simulink subsystem "ButtonStatesRF"  (R2023b)
settings: Solver=VariableStepAuto  StartTime=0.0  StopTime=10.0  RelTol=1e-3
undefined symbols (not defined in the file or in its folder): eStateName
libraries: simulink x5
(part 1 of 2)
settings Model: DataDictionary=ButtonLogicData.sldd  EnableAccessToBaseWorkspace=off  Creator=pkpam  LastModifiedBy=pkpam

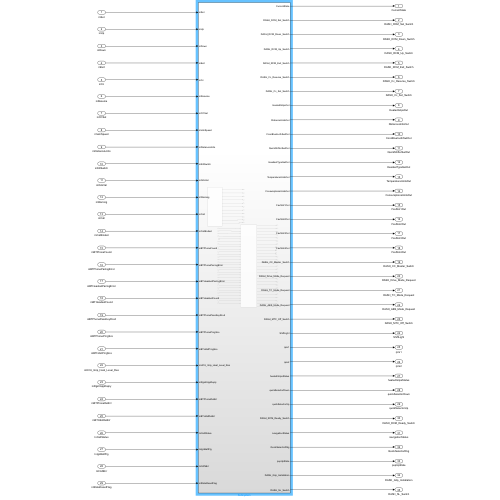
[thumbnail: Simulink's stored preview of the top-level diagram]
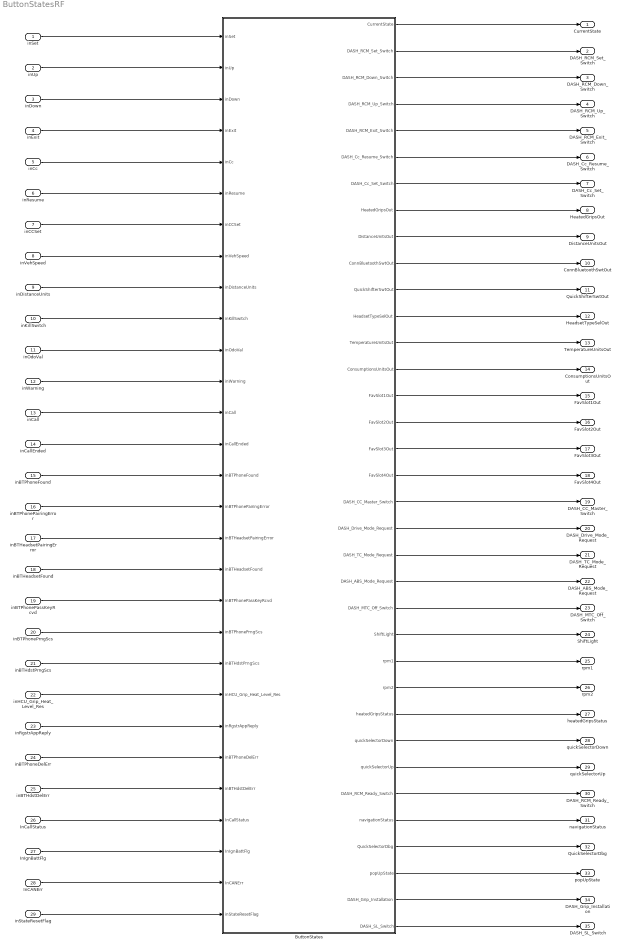
== system ButtonStatesRF ==
  inSet : Inport  OutDataTypeStr=boolean
  inUp : Inport  Port=2  OutDataTypeStr=boolean
  inDown : Inport  Port=3  OutDataTypeStr=boolean
  inExit : Inport  Port=4  OutDataTypeStr=boolean
  inCc : Inport  Port=5
  inResume : Inport  Port=6
  inCCSet : Inport  Port=7
  inVehSpeed : Inport  Port=8  OutDataTypeStr=single
  inDistanceUnits : Inport  Port=9
  inKillSwitch : Inport  Port=10
  inOdoVal : Inport  Port=11
  inWarning : Inport  Port=12  OutDataTypeStr=boolean
  inCall : Inport  Port=13
  inCallEnded : Inport  Port=14  OutDataTypeStr=boolean
  inBTPhoneFound : Inport  Port=15  OutDataTypeStr=boolean
  inBTPhonePairingError : Inport  Port=16  OutDataTypeStr=boolean
  inBTHeadsetPairingError : Inport  Port=17  OutDataTypeStr=boolean
  inBTHeadsetFound : Inport  Port=18  OutDataTypeStr=boolean
  inBTPhonePassKeyRcvd : Inport  Port=19  OutDataTypeStr=boolean
  inBTPhonePrngScs : Inport  Port=20  OutDataTypeStr=boolean
  inBTHdstPrngScs : Inport  Port=21  OutDataTypeStr=boolean
  inHCU_Grip_Heat_Level_Res : Inport  Port=22  OutDataTypeStr=boolean
  inRgstrAppReply : Inport  Port=23  OutDataTypeStr=boolean
  inBTPhoneDelErr : Inport  Port=24  OutDataTypeStr=boolean
  inBTHdstDelErr : Inport  Port=25  OutDataTypeStr=boolean
  InCallStatus : Inport  Port=26  OutDataTypeStr=uint8
  InIgnBattFlg : Inport  Port=27
  InCANErr : Inport  Port=28  OutDataTypeStr=boolean
  inStateResetFlag : Inport  Port=29  OutDataTypeStr=boolean
  ButtonStates : Subsystem
  CurrentState : Outport
  DASH_RCM_Set_Switch : Outport  Port=2
  DASH_RCM_Down_Switch : Outport  Port=3
  DASH_RCM_Up_Switch : Outport  Port=4
  DASH_RCM_Exit_Switch : Outport  Port=5
  DASH_Cc_Resume_Switch : Outport  Port=6
  DASH_Cc_Set_Switch : Outport  Port=7
  HeatedGripsOut : Outport  Port=8
  DistanceUnitsOut : Outport  Port=9
  ConnBluetoothSwtOut : Outport  Port=10
  QuickShifterSwtOut : Outport  Port=11
  HeadsetTypeSelOut : Outport  Port=12
  TemperatureUnitsOut : Outport  Port=13
  ConsumptionsUnitsOut : Outport  Port=14
  FavSlot1Out : Outport  Port=15
  FavSlot2Out : Outport  Port=16
  FavSlot3Out : Outport  Port=17
  FavSlot4Out : Outport  Port=18
  DASH_CC_Master_Switch : Outport  Port=19
  DASH_Drive_Mode_Request : Outport  Port=20
  DASH_TC_Mode_Request : Outport  Port=21
  DASH_ABS_Mode_Request : Outport  Port=22
  DASH_MTC_Off_Switch : Outport  Port=23
  ShiftLight : Outport  Port=24
  rpm1 : Outport  Port=25
  rpm2 : Outport  Port=26
  heatedGripsStatus : Outport  Port=27
  quickSelectorDown : Outport  Port=28
  quickSelectorUp : Outport  Port=29
  DASH_RCM_Ready_Switch : Outport  Port=30
  navigationStatus : Outport  Port=31
  QuickSelectorDbg : Outport  Port=32
  popUpState : Outport  Port=33
  DASH_Grip_Installation : Outport  Port=34
  DASH_SL_Switch : Outport  Port=35  OutDataTypeStr=uint8
  wires:
  ButtonStates/34(DASH_Grip_Installation) -> DASH_Grip_Installation/1
  ButtonStates/33(popUpState) -> popUpState/1
  ButtonStates/32(QuickSelectorDbg) -> QuickSelectorDbg/1
  ButtonStates/30(DASH_RCM_Ready_Switch) -> DASH_RCM_Ready_Switch/1
  ButtonStates/28(quickSelectorDown) -> quickSelectorDown/1
  ButtonStates/27(heatedGripsStatus) -> heatedGripsStatus/1
  ButtonStates/26(rpm2) -> rpm2/1
  ButtonStates/24(ShiftLight) -> ShiftLight/1
  ButtonStates/22(DASH_ABS_Mode_Request) -> DASH_ABS_Mode_Request/1
  ButtonStates/21(DASH_TC_Mode_Request) -> DASH_TC_Mode_Request/1
  ButtonStates/20(DASH_Drive_Mode_Request) -> DASH_Drive_Mode_Request/1
  ButtonStates/16(FavSlot2Out) -> FavSlot2Out/1
  ButtonStates/15(FavSlot1Out) -> FavSlot1Out/1
  ButtonStates/14(ConsumptionsUnitsOut) -> ConsumptionsUnitsOut/1
  ButtonStates/13(TemperatureUnitsOut) -> TemperatureUnitsOut/1
  ButtonStates/12(HeadsetTypeSelOut) -> HeadsetTypeSelOut/1
  ButtonStates/10(ConnBluetoothSwtOut) -> ConnBluetoothSwtOut/1
  ButtonStates/8(HeatedGripsOut) -> HeatedGripsOut/1
  ButtonStates/6(DASH_Cc_Resume_Switch) -> DASH_Cc_Resume_Switch/1
  ButtonStates/4(DASH_RCM_Up_Switch) -> DASH_RCM_Up_Switch/1
  ButtonStates/3(DASH_RCM_Down_Switch) -> DASH_RCM_Down_Switch/1
  ButtonStates/2(DASH_RCM_Set_Switch) -> DASH_RCM_Set_Switch/1
  inBTHdstDelErr/1 -> ButtonStates/25(inBTHdstDelErr)
  inRgstrAppReply/1 -> ButtonStates/23(inRgstrAppReply)
  ButtonStates/9(DistanceUnitsOut) -> DistanceUnitsOut/1
  inHCU_Grip_Heat_Level_Res/1 -> ButtonStates/22(inHCU_Grip_Heat_Level_Res)
  inBTHdstPrngScs/1 -> ButtonStates/21(inBTHdstPrngScs)
  ButtonStates/31(navigationStatus) -> navigationStatus/1
  inBTPhonePrngScs/1 -> ButtonStates/20(inBTPhonePrngScs)
  ButtonStates/11(QuickShifterSwtOut) -> QuickShifterSwtOut/1
  inBTPhonePassKeyRcvd/1 -> ButtonStates/19(inBTPhonePassKeyRcvd)
  inBTHeadsetFound/1 -> ButtonStates/18(inBTHeadsetFound)
  ButtonStates/35(DASH_SL_Switch) -> DASH_SL_Switch/1
  inBTHeadsetPairingError/1 -> ButtonStates/17(inBTHeadsetPairingError)
  ButtonStates/7(DASH_Cc_Set_Switch) -> DASH_Cc_Set_Switch/1
  inBTPhonePairingError/1 -> ButtonStates/16(inBTPhonePairingError)
  inBTPhoneFound/1 -> ButtonStates/15(inBTPhoneFound)
  ButtonStates/29(quickSelectorUp) -> quickSelectorUp/1
  inCallEnded/1 -> ButtonStates/14(inCallEnded)
  inCall/1 -> ButtonStates/13(inCall)
  inWarning/1 -> ButtonStates/12(inWarning)
  ButtonStates/5(DASH_RCM_Exit_Switch) -> DASH_RCM_Exit_Switch/1
  inOdoVal/1 -> ButtonStates/11(inOdoVal)
  InIgnBattFlg/1 -> ButtonStates/27(InIgnBattFlg)
  inKillSwitch/1 -> ButtonStates/10(inKillSwitch)
  ButtonStates/17(FavSlot3Out) -> FavSlot3Out/1
  inDistanceUnits/1 -> ButtonStates/9(inDistanceUnits)
  inStateResetFlag/1 -> ButtonStates/29(inStateResetFlag)
  inCc/1 -> ButtonStates/5(inCc)
  ButtonStates/19(DASH_CC_Master_Switch) -> DASH_CC_Master_Switch/1
  inExit/1 -> ButtonStates/4(inExit)
  inCCSet/1 -> ButtonStates/7(inCCSet)
  inVehSpeed/1 -> ButtonStates/8(inVehSpeed)
  inBTPhoneDelErr/1 -> ButtonStates/24(inBTPhoneDelErr)
  ButtonStates/23(DASH_MTC_Off_Switch) -> DASH_MTC_Off_Switch/1
  inDown/1 -> ButtonStates/3(inDown)
  InCallStatus/1 -> ButtonStates/26(InCallStatus)
  ButtonStates/1(CurrentState) -> CurrentState/1
  inResume/1 -> ButtonStates/6(inResume)
  InCANErr/1 -> ButtonStates/28(InCANErr)
  ButtonStates/25(rpm1) -> rpm1/1
  inSet/1 -> ButtonStates/1(inSet)
  ButtonStates/18(FavSlot4Out) -> FavSlot4Out/1
  inUp/1 -> ButtonStates/2(inUp)

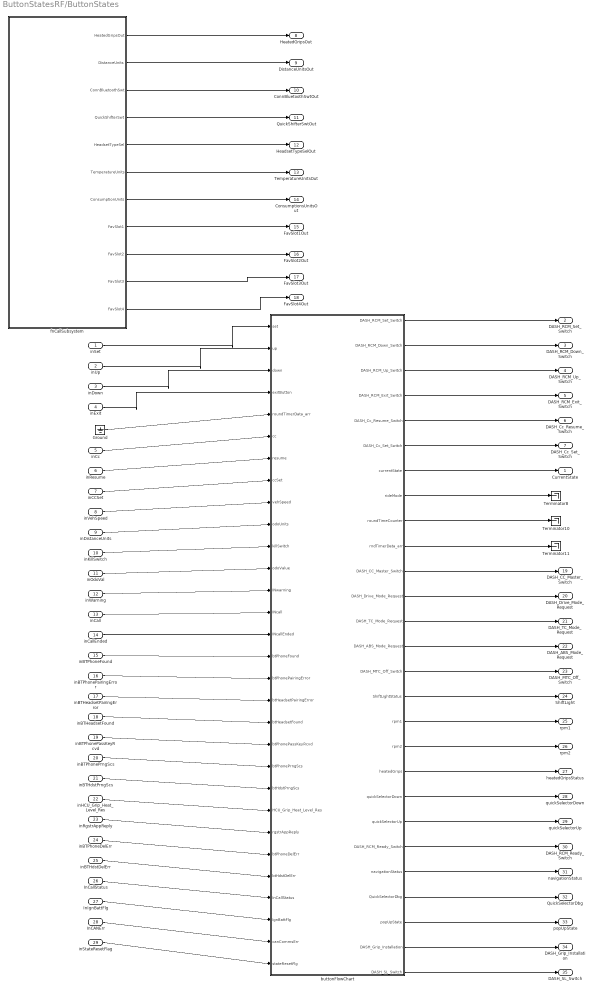
== system ButtonStatesRF/ButtonStates ==
  inSet : Inport  OutDataTypeStr=boolean
  inUp : Inport  Port=2  OutDataTypeStr=boolean
  inDown : Inport  Port=3  OutDataTypeStr=boolean
  inExit : Inport  Port=4  OutDataTypeStr=boolean
  inCc : Inport  Port=5
  inResume : Inport  Port=6
  inCCSet : Inport  Port=7
  inVehSpeed : Inport  Port=8  OutDataTypeStr=single
  inDistanceUnits : Inport  Port=9
  inKillSwitch : Inport  Port=10
  inOdoVal : Inport  Port=11
  inWarning : Inport  Port=12  OutDataTypeStr=boolean
  inCall : Inport  Port=13
  inCallEnded : Inport  Port=14  OutDataTypeStr=boolean
  inBTPhoneFound : Inport  Port=15  OutDataTypeStr=boolean
  inBTPhonePairingError : Inport  Port=16  OutDataTypeStr=boolean
  inBTHeadsetPairingError : Inport  Port=17  OutDataTypeStr=boolean
  inBTHeadsetFound : Inport  Port=18  OutDataTypeStr=boolean
  inBTPhonePassKeyRcvd : Inport  Port=19  OutDataTypeStr=boolean
  inBTPhonePrngScs : Inport  Port=20  OutDataTypeStr=boolean
  inBTHdstPrngScs : Inport  Port=21  OutDataTypeStr=boolean
  inHCU_Grip_Heat_Level_Res : Inport  Port=22  OutDataTypeStr=boolean
  inRgstrAppReply : Inport  Port=23  OutDataTypeStr=boolean
  inBTPhoneDelErr : Inport  Port=24  OutDataTypeStr=boolean
  inBTHdstDelErr : Inport  Port=25  OutDataTypeStr=boolean
  InCallStatus : Inport  Port=26  OutDataTypeStr=uint8
  InIgnBattFlg : Inport  Port=27
  InCANErr : Inport  Port=28  OutDataTypeStr=boolean
  inStateResetFlag : Inport  Port=29  OutDataTypeStr=boolean
  Ground : Ground
  Terminator10 : Terminator
  Terminator11 : Terminator
  Terminator8 : Terminator
  buttonFlowChart : Stateflow chart  TreatAsAtomicUnit=on
  fnCallSubsystem : Subsystem
  CurrentState : Outport
  DASH_RCM_Set_Switch : Outport  Port=2
  DASH_RCM_Down_Switch : Outport  Port=3
  DASH_RCM_Up_Switch : Outport  Port=4
  DASH_RCM_Exit_Switch : Outport  Port=5
  DASH_Cc_Resume_Switch : Outport  Port=6
  DASH_Cc_Set_Switch : Outport  Port=7
  HeatedGripsOut : Outport  Port=8
  DistanceUnitsOut : Outport  Port=9
  ConnBluetoothSwtOut : Outport  Port=10
  QuickShifterSwtOut : Outport  Port=11
  HeadsetTypeSelOut : Outport  Port=12
  TemperatureUnitsOut : Outport  Port=13
  ConsumptionsUnitsOut : Outport  Port=14
  FavSlot1Out : Outport  Port=15
  FavSlot2Out : Outport  Port=16
  FavSlot3Out : Outport  Port=17
  FavSlot4Out : Outport  Port=18
  DASH_CC_Master_Switch : Outport  Port=19
  DASH_Drive_Mode_Request : Outport  Port=20
  DASH_TC_Mode_Request : Outport  Port=21
  DASH_ABS_Mode_Request : Outport  Port=22
  DASH_MTC_Off_Switch : Outport  Port=23
  ShiftLight : Outport  Port=24
  rpm1 : Outport  Port=25
  rpm2 : Outport  Port=26
  heatedGripsStatus : Outport  Port=27
  quickSelectorDown : Outport  Port=28
  quickSelectorUp : Outport  Port=29
  DASH_RCM_Ready_Switch : Outport  Port=30
  navigationStatus : Outport  Port=31
  QuickSelectorDbg : Outport  Port=32
  popUpState : Outport  Port=33
  DASH_Grip_Installation : Outport  Port=34
  DASH_SL_Switch : Outport  Port=35  OutDataTypeStr=uint8
  wires:
  buttonFlowChart/10(rndTimerData_arr) -> Terminator11/1
  fnCallSubsystem/7(ConsumptionUnits) -> ConsumptionsUnitsOut/1
  inHCU_Grip_Heat_Level_Res/1 -> buttonFlowChart/23(HCU_Grip_Heat_Level_Res)
  fnCallSubsystem/1(HeatedGripsOut) -> HeatedGripsOut/1
  buttonFlowChart/22(DASH_RCM_Ready_Switch) -> DASH_RCM_Ready_Switch/1
  buttonFlowChart/19(heatedGrips) -> heatedGripsStatus/1
  buttonFlowChart/8(rideMode) -> Terminator8/1
  buttonFlowChart/17(rpm1) -> rpm1/1
  buttonFlowChart/21(quickSelectorUp) -> quickSelectorUp/1
  buttonFlowChart/24(QuickSelectorDbg) -> QuickSelectorDbg/1
  inBTHeadsetFound/1 -> buttonFlowChart/19(btHeadsetFound)
  inCall/1 -> buttonFlowChart/14(INcall)
  buttonFlowChart/7(currentState) -> CurrentState/1
  fnCallSubsystem/6(TemperatureUnits) -> TemperatureUnitsOut/1
  buttonFlowChart/4(DASH_RCM_Exit_Switch) -> DASH_RCM_Exit_Switch/1
  inUp/1 -> buttonFlowChart/2(up)
  inBTPhonePassKeyRcvd/1 -> buttonFlowChart/20(btPhonePassKeyRcvd)
  inResume/1 -> buttonFlowChart/7(resume)
  buttonFlowChart/9(roundTimeCounter) -> Terminator10/1
  InIgnBattFlg/1 -> buttonFlowChart/28(IgnBattFlg)
  buttonFlowChart/18(rpm2) -> rpm2/1
  Ground/1 -> buttonFlowChart/5(roundTimerData_arr)
  inOdoVal/1 -> buttonFlowChart/12(odoValue)
  fnCallSubsystem/8(FavSlot1) -> FavSlot1Out/1
  fnCallSubsystem/9(FavSlot2) -> FavSlot2Out/1
  inBTPhoneDelErr/1 -> buttonFlowChart/25(btPhoneDelErr)
  inBTPhonePrngScs/1 -> buttonFlowChart/21(btPhonePrngScs)
  inCallEnded/1 -> buttonFlowChart/15(INcallEnded)
  buttonFlowChart/14(DASH_ABS_Mode_Request) -> DASH_ABS_Mode_Request/1
  buttonFlowChart/12(DASH_Drive_Mode_Request) -> DASH_Drive_Mode_Request/1
  InCallStatus/1 -> buttonFlowChart/27(InCallStatus)
  buttonFlowChart/3(DASH_RCM_Up_Switch) -> DASH_RCM_Up_Switch/1
  inWarning/1 -> buttonFlowChart/13(INwarning)
  buttonFlowChart/23(navigationStatus) -> navigationStatus/1
  inCCSet/1 -> buttonFlowChart/8(ccSet)
  buttonFlowChart/1(DASH_RCM_Set_Switch) -> DASH_RCM_Set_Switch/1
  inSet/1 -> buttonFlowChart/1(set)
  buttonFlowChart/6(DASH_Cc_Set_Switch) -> DASH_Cc_Set_Switch/1
  buttonFlowChart/5(DASH_Cc_Resume_Switch) -> DASH_Cc_Resume_Switch/1
  fnCallSubsystem/11(FavSlot4) -> FavSlot4Out/1
  inCc/1 -> buttonFlowChart/6(cc)
  fnCallSubsystem/10(FavSlot3) -> FavSlot3Out/1
  inDistanceUnits/1 -> buttonFlowChart/10(odoUnits)
  buttonFlowChart/27(DASH_SL_Switch) -> DASH_SL_Switch/1
  inDown/1 -> buttonFlowChart/3(down)
  buttonFlowChart/15(DASH_MTC_Off_Switch) -> DASH_MTC_Off_Switch/1
  inBTPhonePairingError/1 -> buttonFlowChart/17(btPhonePairingError)
  buttonFlowChart/26(DASH_Grip_Installation) -> DASH_Grip_Installation/1
  inBTHeadsetPairingError/1 -> buttonFlowChart/18(btHeadsetPairingError)
  inBTHdstDelErr/1 -> buttonFlowChart/26(btHdstDelErr)
  inExit/1 -> buttonFlowChart/4(exitButton)
  inBTPhoneFound/1 -> buttonFlowChart/16(btPhoneFound)
  InCANErr/1 -> buttonFlowChart/29(canCommsErr)
  fnCallSubsystem/5(HeadsetTypeSel) -> HeadsetTypeSelOut/1
  buttonFlowChart/25(popUpState) -> popUpState/1
  fnCallSubsystem/3(ConnBluetoothSwt) -> ConnBluetoothSwtOut/1
  inStateResetFlag/1 -> buttonFlowChart/30(stateResetFlg)
  buttonFlowChart/13(DASH_TC_Mode_Request) -> DASH_TC_Mode_Request/1
  inVehSpeed/1 -> buttonFlowChart/9(vehSpeed)
  inRgstrAppReply/1 -> buttonFlowChart/24(rgstrAppReply)
  inBTHdstPrngScs/1 -> buttonFlowChart/22(btHdstPrngScs)
  buttonFlowChart/20(quickSelectorDown) -> quickSelectorDown/1
  buttonFlowChart/11(DASH_CC_Master_Switch) -> DASH_CC_Master_Switch/1
  inKillSwitch/1 -> buttonFlowChart/11(killSwitch)
  fnCallSubsystem/2(DistanceUnits) -> DistanceUnitsOut/1
  buttonFlowChart/16(ShiftLightStatus) -> ShiftLight/1
  buttonFlowChart/2(DASH_RCM_Down_Switch) -> DASH_RCM_Down_Switch/1
  fnCallSubsystem/4(QuickShifterSwt) -> QuickShifterSwtOut/1

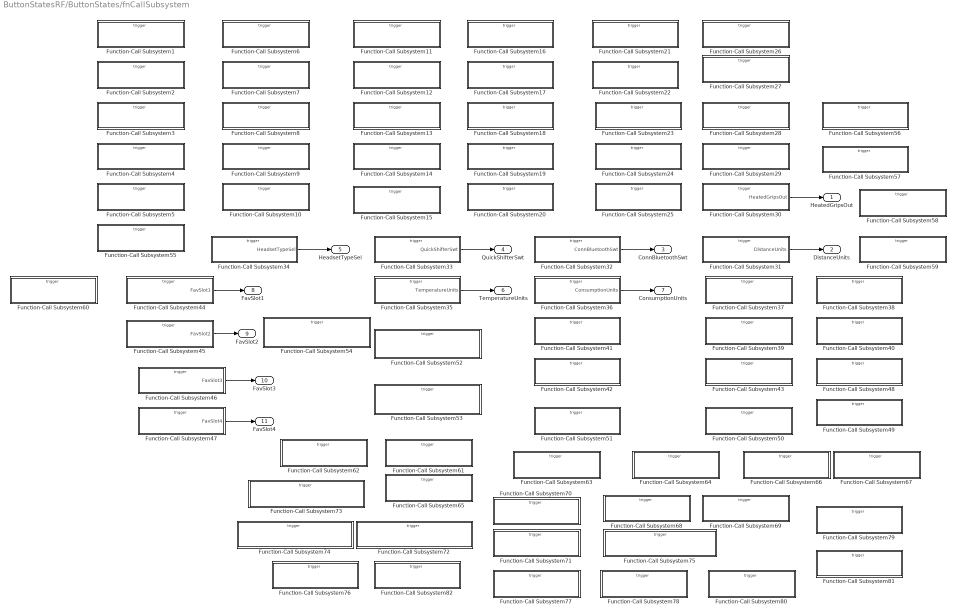
== system ButtonStatesRF/ButtonStates/fnCallSubsystem ==
  Function-Call Subsystem1 : Subsystem  TreatAsAtomicUnit=on
  Function-Call Subsystem10 : Subsystem  TreatAsAtomicUnit=on
  Function-Call Subsystem11 : Subsystem  TreatAsAtomicUnit=on
  Function-Call Subsystem12 : Subsystem  TreatAsAtomicUnit=on
  Function-Call Subsystem13 : Subsystem  TreatAsAtomicUnit=on
  Function-Call Subsystem14 : Subsystem  TreatAsAtomicUnit=on
  Function-Call Subsystem15 : Subsystem  TreatAsAtomicUnit=on
  Function-Call Subsystem16 : Subsystem  TreatAsAtomicUnit=on
  Function-Call Subsystem17 : Subsystem  TreatAsAtomicUnit=on
  Function-Call Subsystem18 : Subsystem  TreatAsAtomicUnit=on
  Function-Call Subsystem19 : Subsystem  TreatAsAtomicUnit=on
  Function-Call Subsystem2 : Subsystem  TreatAsAtomicUnit=on
  Function-Call Subsystem20 : Subsystem  TreatAsAtomicUnit=on
  Function-Call Subsystem21 : Subsystem  TreatAsAtomicUnit=on
  Function-Call Subsystem22 : Subsystem  TreatAsAtomicUnit=on
  Function-Call Subsystem23 : Subsystem  TreatAsAtomicUnit=on
  Function-Call Subsystem24 : Subsystem  TreatAsAtomicUnit=on
  Function-Call Subsystem25 : Subsystem  TreatAsAtomicUnit=on
  Function-Call Subsystem26 : Subsystem  TreatAsAtomicUnit=on
  Function-Call Subsystem27 : Subsystem  TreatAsAtomicUnit=on
  Function-Call Subsystem28 : Subsystem  TreatAsAtomicUnit=on
  Function-Call Subsystem29 : Subsystem  TreatAsAtomicUnit=on
  Function-Call Subsystem3 : Subsystem  TreatAsAtomicUnit=on
  Function-Call Subsystem30 : Subsystem  TreatAsAtomicUnit=on
  Function-Call Subsystem31 : Subsystem  TreatAsAtomicUnit=on
  Function-Call Subsystem32 : Subsystem  TreatAsAtomicUnit=on
  Function-Call Subsystem33 : Subsystem  TreatAsAtomicUnit=on
  Function-Call Subsystem34 : Subsystem  TreatAsAtomicUnit=on
  Function-Call Subsystem35 : Subsystem  TreatAsAtomicUnit=on
  Function-Call Subsystem36 : Subsystem  TreatAsAtomicUnit=on
  Function-Call Subsystem37 : Subsystem  TreatAsAtomicUnit=on
  Function-Call Subsystem38 : Subsystem  TreatAsAtomicUnit=on
  Function-Call Subsystem39 : Subsystem  TreatAsAtomicUnit=on
  Function-Call Subsystem4 : Subsystem  TreatAsAtomicUnit=on
  Function-Call Subsystem40 : Subsystem  TreatAsAtomicUnit=on
  Function-Call Subsystem41 : Subsystem  TreatAsAtomicUnit=on
  Function-Call Subsystem42 : Subsystem  TreatAsAtomicUnit=on
  Function-Call Subsystem43 : Subsystem  TreatAsAtomicUnit=on
  Function-Call Subsystem44 : Subsystem  TreatAsAtomicUnit=on
  Function-Call Subsystem45 : Subsystem  TreatAsAtomicUnit=on
  Function-Call Subsystem46 : Subsystem  TreatAsAtomicUnit=on
  Function-Call Subsystem47 : Subsystem  TreatAsAtomicUnit=on
  Function-Call Subsystem48 : Subsystem  TreatAsAtomicUnit=on
  Function-Call Subsystem49 : Subsystem  TreatAsAtomicUnit=on
  Function-Call Subsystem5 : Subsystem  TreatAsAtomicUnit=on
  Function-Call Subsystem50 : Subsystem  TreatAsAtomicUnit=on
  Function-Call Subsystem51 : Subsystem  TreatAsAtomicUnit=on
  Function-Call Subsystem52 : Subsystem  TreatAsAtomicUnit=on
  Function-Call Subsystem53 : Subsystem  TreatAsAtomicUnit=on
  Function-Call Subsystem54 : Subsystem  TreatAsAtomicUnit=on
  Function-Call Subsystem55 : Subsystem  TreatAsAtomicUnit=on
  Function-Call Subsystem56 : Subsystem  TreatAsAtomicUnit=on
  Function-Call Subsystem57 : Subsystem  TreatAsAtomicUnit=on
  Function-Call Subsystem58 : Subsystem  TreatAsAtomicUnit=on
  Function-Call Subsystem59 : Subsystem  TreatAsAtomicUnit=on
  Function-Call Subsystem6 : Subsystem  TreatAsAtomicUnit=on
  Function-Call Subsystem60 : Subsystem  TreatAsAtomicUnit=on
  Function-Call Subsystem61 : Subsystem  TreatAsAtomicUnit=on
  Function-Call Subsystem62 : Subsystem  TreatAsAtomicUnit=on
  Function-Call Subsystem63 : Subsystem  TreatAsAtomicUnit=on
  Function-Call Subsystem64 : Subsystem  TreatAsAtomicUnit=on
  Function-Call Subsystem65 : Subsystem  TreatAsAtomicUnit=on
  Function-Call Subsystem66 : Subsystem  TreatAsAtomicUnit=on
  Function-Call Subsystem67 : Subsystem  TreatAsAtomicUnit=on
  Function-Call Subsystem68 : Subsystem  TreatAsAtomicUnit=on
  Function-Call Subsystem69 : Subsystem  TreatAsAtomicUnit=on
  Function-Call Subsystem7 : Subsystem  TreatAsAtomicUnit=on
  Function-Call Subsystem70 : Subsystem  TreatAsAtomicUnit=on
  Function-Call Subsystem71 : Subsystem  TreatAsAtomicUnit=on
  Function-Call Subsystem72 : Subsystem  TreatAsAtomicUnit=on
  Function-Call Subsystem73 : Subsystem  TreatAsAtomicUnit=on
  Function-Call Subsystem74 : Subsystem  TreatAsAtomicUnit=on
  Function-Call Subsystem75 : Subsystem  TreatAsAtomicUnit=on
  Function-Call Subsystem76 : Subsystem  TreatAsAtomicUnit=on
  Function-Call Subsystem77 : Subsystem  TreatAsAtomicUnit=on
  Function-Call Subsystem78 : Subsystem  TreatAsAtomicUnit=on
  Function-Call Subsystem79 : Subsystem  TreatAsAtomicUnit=on
  Function-Call Subsystem8 : Subsystem  TreatAsAtomicUnit=on
  Function-Call Subsystem80 : Subsystem  TreatAsAtomicUnit=on
  Function-Call Subsystem81 : Subsystem  TreatAsAtomicUnit=on
  Function-Call Subsystem82 : Subsystem  TreatAsAtomicUnit=on
  Function-Call Subsystem9 : Subsystem  TreatAsAtomicUnit=on
  HeatedGripsOut : Outport
  DistanceUnits : Outport  Port=2
  ConnBluetoothSwt : Outport  Port=3
  QuickShifterSwt : Outport  Port=4
  HeadsetTypeSel : Outport  Port=5
  TemperatureUnits : Outport  Port=6
  ConsumptionUnits : Outport  Port=7
  FavSlot1 : Outport  Port=8
  FavSlot2 : Outport  Port=9
  FavSlot3 : Outport  Port=10
  FavSlot4 : Outport  Port=11
  wires:
  Function-Call Subsystem30/1(HeatedGripsOut) -> HeatedGripsOut/1
  Function-Call Subsystem31/1(DistanceUnits) -> DistanceUnits/1
  Function-Call Subsystem32/1(ConnBluetoothSwt) -> ConnBluetoothSwt/1
  Function-Call Subsystem33/1(QuickShifterSwt) -> QuickShifterSwt/1
  Function-Call Subsystem34/1(HeadsetTypeSel) -> HeadsetTypeSel/1
  Function-Call Subsystem35/1(TemperatureUnits) -> TemperatureUnits/1
  Function-Call Subsystem36/1(ConsumptionUnits) -> ConsumptionUnits/1
  Function-Call Subsystem44/1(FavSlot1) -> FavSlot1/1
  Function-Call Subsystem45/1(FavSlot2) -> FavSlot2/1
  Function-Call Subsystem46/1(FavSlot3) -> FavSlot3/1
  Function-Call Subsystem47/1(FavSlot4) -> FavSlot4/1

== system ButtonStatesRF/ButtonStates/fnCallSubsystem/Function-Call Subsystem1 ==
  BLU_FnRLRndTmrUp : TriggerPort  TriggerType=function-call  IsSimulinkFunction=on  FunctionName=BLU_FnRLRndTmrUp  VariantControl=(inherit)

== system ButtonStatesRF/ButtonStates/fnCallSubsystem/Function-Call Subsystem10 ==
  BLU_FnCCSwtONCrsCtrl : TriggerPort  TriggerType=function-call  IsSimulinkFunction=on  FunctionName=BLU_FnCCSwtONCrsCtrl  VariantControl=(inherit)

== system ButtonStatesRF/ButtonStates/fnCallSubsystem/Function-Call Subsystem11 ==
  BLU_FnAudioVolUp : TriggerPort  TriggerType=function-call  IsSimulinkFunction=on  FunctionName=BLU_FnAudioVolUp  VariantControl=(inherit)

== system ButtonStatesRF/ButtonStates/fnCallSubsystem/Function-Call Subsystem12 ==
  BLU_FnAudioVolDwn : TriggerPort  TriggerType=function-call  IsSimulinkFunction=on  FunctionName=BLU_FnAudioVolDwn  VariantControl=(inherit)

== system ButtonStatesRF/ButtonStates/fnCallSubsystem/Function-Call Subsystem13 ==
  BLU_FnAudioTglPlayPause : TriggerPort  TriggerType=function-call  IsSimulinkFunction=on  FunctionName=BLU_FnAudioTglPlayPause  VariantControl=(inherit)

== system ButtonStatesRF/ButtonStates/fnCallSubsystem/Function-Call Subsystem14 ==
  BLU_FnAudioPrevTrack : TriggerPort  TriggerType=function-call  IsSimulinkFunction=on  FunctionName=BLU_FnAudioPrevTrack  VariantControl=(inherit)

== system ButtonStatesRF/ButtonStates/fnCallSubsystem/Function-Call Subsystem15 ==
  BLU_FnAudioNextTrack : TriggerPort  TriggerType=function-call  IsSimulinkFunction=on  FunctionName=BLU_FnAudioNextTrack  VariantControl=(inherit)

== system ButtonStatesRF/ButtonStates/fnCallSubsystem/Function-Call Subsystem16 ==
  BLU_FnNavVolUp : TriggerPort  TriggerType=function-call  IsSimulinkFunction=on  FunctionName=BLU_FnNavVolUp  VariantControl=(inherit)

== system ButtonStatesRF/ButtonStates/fnCallSubsystem/Function-Call Subsystem17 ==
  BLU_FnNavVolDwn : TriggerPort  TriggerType=function-call  IsSimulinkFunction=on  FunctionName=BLU_FnNavVolDwn  VariantControl=(inherit)

== system ButtonStatesRF/ButtonStates/fnCallSubsystem/Function-Call Subsystem18 ==
  BLU_FnClkSaveTime : TriggerPort  TriggerType=function-call  IsSimulinkFunction=on  FunctionName=BLU_FnClkSaveTime  VariantControl=(inherit)

== system ButtonStatesRF/ButtonStates/fnCallSubsystem/Function-Call Subsystem19 ==
  BLU_FnClkIncHrs : TriggerPort  TriggerType=function-call  IsSimulinkFunction=on  FunctionName=BLU_FnClkIncHrs  VariantControl=(inherit)
  Terminator : Terminator
  u : ArgIn  ArgumentName=u
  wires:
  u/1 -> Terminator/1

== system ButtonStatesRF/ButtonStates/fnCallSubsystem/Function-Call Subsystem2 ==
  BLU_FnRLRndTmrDwn : TriggerPort  TriggerType=function-call  IsSimulinkFunction=on  FunctionName=BLU_FnRLRndTmrDwn  VariantControl=(inherit)

== system ButtonStatesRF/ButtonStates/fnCallSubsystem/Function-Call Subsystem20 ==
  BLU_FnClkDecHrs : TriggerPort  TriggerType=function-call  IsSimulinkFunction=on  FunctionName=BLU_FnClkDecHrs  VariantControl=(inherit)
  Terminator : Terminator
  u : ArgIn  ArgumentName=u
  wires:
  u/1 -> Terminator/1

== system ButtonStatesRF/ButtonStates/fnCallSubsystem/Function-Call Subsystem21 ==
  BLU_FnClkIncMins : TriggerPort  TriggerType=function-call  IsSimulinkFunction=on  FunctionName=BLU_FnClkIncMins  VariantControl=(inherit)
  Terminator : Terminator
  u : ArgIn  ArgumentName=u
  wires:
  u/1 -> Terminator/1

== system ButtonStatesRF/ButtonStates/fnCallSubsystem/Function-Call Subsystem22 ==
  BLU_FnClkDecMins : TriggerPort  TriggerType=function-call  IsSimulinkFunction=on  FunctionName=BLU_FnClkDecMins  VariantControl=(inherit)
  Terminator : Terminator
  u : ArgIn  ArgumentName=u
  wires:
  u/1 -> Terminator/1

== system ButtonStatesRF/ButtonStates/fnCallSubsystem/Function-Call Subsystem23 ==
  BLU_FnClkSaveDate : TriggerPort  TriggerType=function-call  IsSimulinkFunction=on  FunctionName=BLU_FnClkSaveDate  VariantControl=(inherit)